simulink model "CCMLin2Mgr"  (R2022b)
settings: Solver=FixedStepDiscrete  StartTime=0.0  StopTime=10.0  FixedStep=0.02  RelTol=1e-3
undefined symbols (not defined in the file or in its folder): ComChlType, NetworkChlType, P_LinIfSchHandleType, P_Std_ReturnType
(part 3 of 3)

== system CCMLin2Mgr/Lin2ParaService/Lin2ParaService_ReadVbmrPercent_sys ==
  function : TriggerPort  TriggerType=function-call  IsSimulinkFunction=on  FunctionName=Lin2ParaService_ReadVbmrPercent  VariantControl=(inherit)
  Ground_1 : Ground
  Ground_2 : Ground
  Percent : ArgOut  OutDataTypeStr=UInt8  ArgumentName=Percent
  ERR : ArgOut  Port=2  OutDataTypeStr=Std_ReturnType  ArgumentName=ERR
  wires:
  Ground_1/1 -> Percent/1
  Ground_2/1 -> ERR/1

== system CCMLin2Mgr/Lin2ParaService/Lin2ParaService_VbmrReqPercentRetCtrltoECU_sys ==
  function : TriggerPort  TriggerType=function-call  IsSimulinkFunction=on  FunctionName=Lin2ParaService_VbmrReqPercentRetCtrltoECU  VariantControl=(inherit)
  Ground_1 : Ground
  ERR : ArgOut  OutDataTypeStr=Std_ReturnType  ArgumentName=ERR
  wires:
  Ground_1/1 -> ERR/1

== system CCMLin2Mgr/Lin2ParaService/Lin2ParaService_VbmrReqPercentShortTermAdj_sys ==
  function : TriggerPort  TriggerType=function-call  IsSimulinkFunction=on  FunctionName=Lin2ParaService_VbmrReqPercentShortTermAdj  VariantControl=(inherit)
  Ground_1 : Ground
  Terminator_1 : Terminator
  Percent : ArgIn  OutDataTypeStr=UInt8  ArgumentName=Percent
  ERR : ArgOut  OutDataTypeStr=Std_ReturnType  ArgumentName=ERR
  wires:
  Percent/1 -> Terminator_1/1
  Ground_1/1 -> ERR/1
